simulink model "gate_V37_2016a_HIL_C"  (R2016a)
settings: Solver=FixedStepDiscrete  StartTime=0.0  StopTime=120  FixedStep=0.001  RelTol=1e-3
values from neighbouring files (symbols this model uses):
  CLOSE_SIDE_VALUE = single(4*ONE_SEC)    [GateParameter.m]
  CLOSED = uint8(12)    [GateParameter.m]
  CLOSING = uint8(13)    [GateParameter.m]
  CURRENT_STABLE_TIMER = uint8(200)    [GateParameter.m]
  CURRENT_THRESH = uint16(1000)    [GateParameter.m]
  INIT = uint8(15)    [GateParameter.m]
  MOVING_TIMER = uint32(60*ONE_SEC)    [GateParameter.m]
  OPEN = uint8(14)    [GateParameter.m]
  OPEN_SIDE_VALUE = uint16(4*ONE_SEC)    [GateParameter.m]
  OPENING = uint8(11)    [GateParameter.m]
  OPENING_ACTIVE = uint8(1)    [GateParameter.m]
  STABLE_TIMER_VALUE = uint16(1*ONE_SEC)    [GateParameter.m]
undefined symbols (not defined in the file or in its folder): sigbuilder_block
libraries: simulink x9, arduinolib x7
(part 1 of 2)
settings Model: ExtModeTrigDuration=2  Creator=omaro  LastModifiedBy=oelkawok
settings DataIOCC: SaveFormat=StructureWithTime  SaveOutput=off  SaveTime=off
settings OptimizationCC: StateBitsets=on  DataBitsets=on  LifeSpan=1  MaxStackSize=64
settings DebuggingCC: ConsistencyChecking=error  SignalInfNanChecking=error  SignalRangeChecking=error  ReadBeforeWriteMsg=EnableAllAsError  WriteAfterWriteMsg=EnableAllAsError  WriteAfterReadMsg=EnableAllAsError  AlgebraicLoopMsg=error  ArtificialAlgebraicLoopMsg=error  BlockPriorityViolationMsg=error  MinStepSizeMsg=error  CheckMatrixSingularityMsg=error  ParameterUnderflowMsg=error  ParameterPrecisionLossMsg=error  UnderSpecifiedDataTypeMsg=warning  UnnecessaryDatatypeConvMsg=warning  VectorMatrixConversionMsg=error  SignalLabelMismatchMsg=error  UnconnectedInputMsg=error  UnconnectedOutputMsg=error  UnconnectedLineMsg=error  SFcnCompatibilityMsg=error  UniqueDataStoreMsg=error  BusObjectLabelMismatch=error  RootOutportRequireBusObject=error  ModelReferenceIOMsg=error  ModelReferenceVersionMismatchMessage=warning  ModelReferenceIOMismatchMessage=error  ModelReferenceDataLoggingMessage=error  StrictBusMsg=ErrorOnBusTreatedAsVector  SFUnusedDataAndEventsDiag=error  SFUnexpectedBacktrackingDiag=error  SFInvalidInputDataAccessInChartInitDiag=error  SFNoUnconditionalDefaultTransitionDiag=error  SFTransitionOutsideNaturalParentDiag=error  SFUndirectedBroadcastEventsDiag=error  SFTransitionActionBeforeConditionDiag=error  SFOutputUsedAsStateInMooreChartDiag=warning
settings HardwareCC: ProdBitPerInt=16  ProdBitPerPointer=16  ProdLargestAtomicFloat=None  ProdWordSize=8  ProdHWDeviceType=Atmel->AVR
settings SFSimCC: SimCustomHeaderCode=|
  | //#include "C:\Users\omaro\Desktop\Gate_Model\Arduino\Arduino.h"
  | //#include "Keypad\Keypad.h"
settings RTWCC: SystemTargetFile=ert.tlc  HardwareBoard=Arduino Nano 3.0  TemplateMakefile=ert_default_tmf  GenerateReport=on  RTWUseSimCustomCode=on  Toolchain=Arduino AVR v1.6.1  BuildConfiguration=Faster Runs  LaunchReport=on
settings RTWCC: CustomHeaderCode=|
  | //#include "C:\Users\omaro\Desktop\Gate_Model\Arduino\Arduino.h"
  | //#include "Keypad\Keypad.h"
settings CodeAppCC: ForceParamTrailComments=on  OperatorAnnotations=on  MATLABSourceComments=on
settings ERTTargetCC: ERTCustomFileBanners=off  SupportContinuousTime=on  SupportNonInlinedSFcns=on  SupportVariableSizeSignals=on  ModelStepFunctionPrototypeControlCompliant=off  RootIOFormat=Structure reference
settings ConfigComp: CovEnable=on  CovScope=ReferencedModels  CovIncludeTopModel=off  CovPath=Gate  CovMetricSettings=dcw  CovHTMLOptions=-sRT=1 -sVT=0 -aTS=1 -bRG=1 -bTC=1 -hTR=0 -nFC=0 -nFD=1 -scm=1 -bcm=1 -xEv=1 -xEM=0  CovHtmlReporting=off  CovModelRefEnable=all

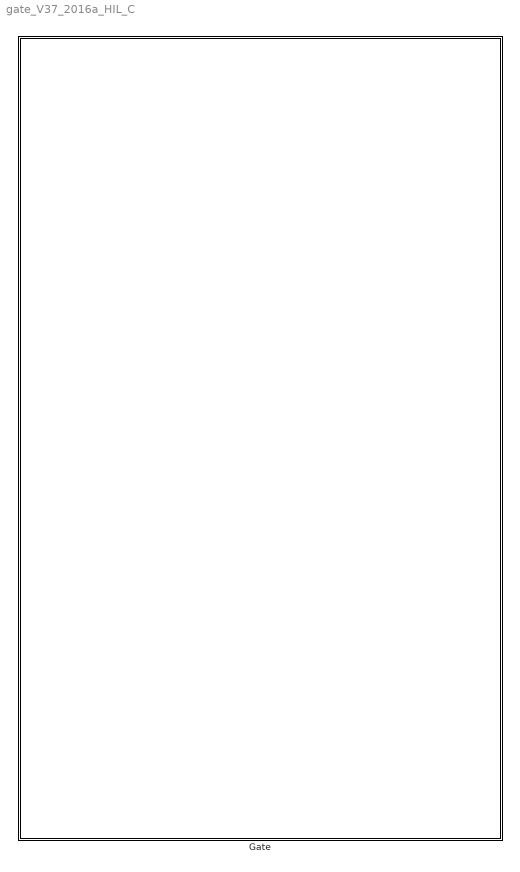
== system gate_V37_2016a_HIL_C ==
  Gate : Subsystem

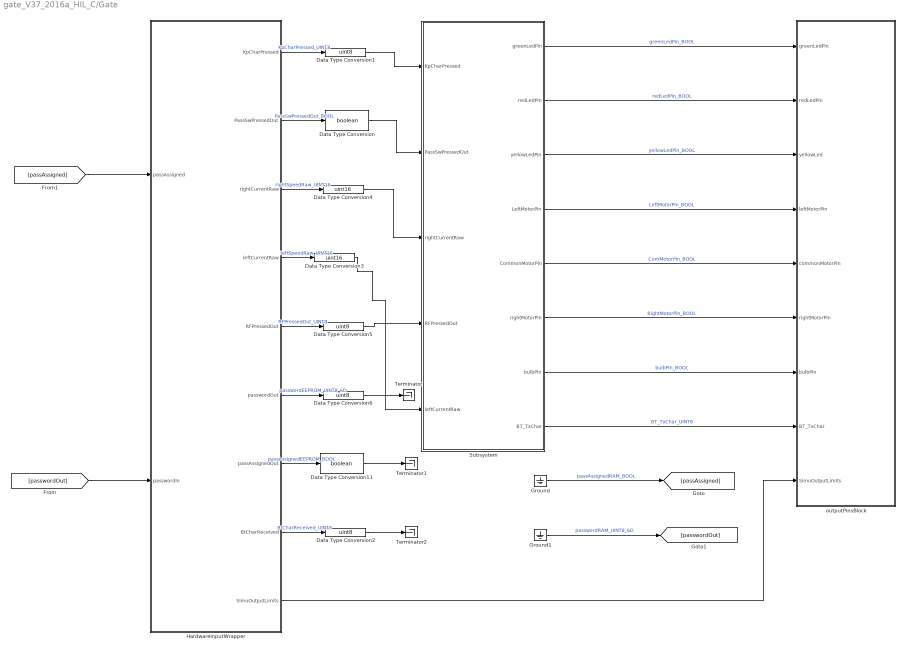
== system gate_V37_2016a_HIL_C/Gate ==
  Data Type Conversion : DataTypeConversion  OutDataTypeStr=boolean
  Data Type Conversion{1..2,5..6} (4 alike) : DataTypeConversion  OutDataTypeStr=uint8
  Data Type Conversion11 : DataTypeConversion  OutDataTypeStr=boolean
  Data Type Conversion3 : DataTypeConversion  OutDataTypeStr=uint16
  Data Type Conversion4 : DataTypeConversion  OutDataTypeStr=uint16
  Ground : Ground
  Ground1 : Ground
  HardwareInputWrapper : Subsystem  Variant=on  VariantControl=Variant1  OverrideUsingVariant=HardwareInloopInputs_C
  Subsystem : Subsystem
  Terminator : Terminator
  Terminator1 : Terminator
  Terminator2 : Terminator
  outputPinsBlock : Subsystem  Variant=on  VariantControl=Variant1  OverrideUsingVariant=HardwareInLoopOutputs_C
  wires:
  HardwareInputWrapper/9(SimuOutputLimits) -> outputPinsBlock/9(SimuOutputLimits)
  HardwareInputWrapper/8(BtCharReceived) -> Data Type Conversion2/1  "BtCharReceived_UINT8"
  HardwareInputWrapper/2(PassSwPressedOut) -> Data Type Conversion/1  "PassSwPressedOut_BOOL"
  HardwareInputWrapper/3(rightCurrentRaw) -> Data Type Conversion4/1  "rightSpeedRaw_UINT16"
  HardwareInputWrapper/5(RFPressedOut) -> Data Type Conversion5/1  "RFPressedOut_UINT8"
  Subsystem/1(greenLedPin) -> outputPinsBlock/1(greenLedPin)  "greenLedPin_BOOL"
  Subsystem/2(redLedPin) -> outputPinsBlock/2(redLedPin)  "redLedPin_BOOL"
  Subsystem/3(yellowLedPin) -> outputPinsBlock/3(yellowLed)  "yellowLedPin_BOOL"
  Subsystem/4(LeftMotorPin) -> outputPinsBlock/4(leftMotorPin)  "LeftMotorPin_BOOL"
  Subsystem/5(CommonMotorPin) -> outputPinsBlock/5(commonMotorPin)  "ComMotorPin_BOOL"
  Subsystem/6(rightMotorPin) -> outputPinsBlock/6(rightMotorPin)  "RightMotorPin_BOOL"
  Subsystem/7(bulbPin) -> outputPinsBlock/7(bulbPin)  "bulbPin_BOOL"
  Subsystem/8(BT_TxChar) -> outputPinsBlock/8(BT_TxChar)  "BT_TxChar_UINT8"
  HardwareInputWrapper/4(leftCurrentRaw) -> Data Type Conversion3/1  "leftSpeedRaw_UINT16"
  HardwareInputWrapper/1(KpCharPressed) -> Data Type Conversion1/1  "KpCharPressed_UINT8"
  Ground1/1 -> [passwordOut]  "passwordRAM_UINT8_6D"
  Ground/1 -> [passAssigned]  "passAssignedRAM_BOOL"
  [passwordOut] -> HardwareInputWrapper/2(passwordIn)
  [passAssigned] -> HardwareInputWrapper/1(passAssigned)
  HardwareInputWrapper/6(passwordOut) -> Data Type Conversion6/1  "passwordEEPROM_UINT8_6D"
  HardwareInputWrapper/7(passAssignedOut) -> Data Type Conversion11/1  "passAssignedEEPROM_BOOL"
  Data Type Conversion1/1 -> Subsystem/1(KpCharPressed)
  Data Type Conversion/1 -> Subsystem/2(PassSwPressedOut)
  Data Type Conversion4/1 -> Subsystem/3(rightCurrentRaw)
  Data Type Conversion3/1 -> Subsystem/5(leftCurrentRaw)
  Data Type Conversion5/1 -> Subsystem/4(RFPressedOut)
  Data Type Conversion11/1 -> Terminator1/1
  Data Type Conversion6/1 -> Terminator/1
  Data Type Conversion2/1 -> Terminator2/1

== system gate_V37_2016a_HIL_C/Gate/HardwareInputWrapper ==
  passAssigned : Inport
  passwordIn : Inport  Port=2
  HIL_B : Subsystem  VariantControl=HardwareInloopInputs_B
  HIL_C : Subsystem  VariantControl=HardwareInloopInputs_C
  MIL_Gate_A : Subsystem  VariantControl=MIL_VariantA
  MIL_Gate_B : Subsystem  VariantControl=MIL_VariantB
  MIL_Gate_C : Subsystem  VariantControl=MIL_VariantC
  KpCharPressed : Outport
  PassSwPressedOut : Outport  Port=2
  rightCurrentRaw : Outport  Port=3
  leftCurrentRaw : Outport  Port=4
  RFPressedOut : Outport  Port=5
  passwordOut : Outport  Port=6
  passAssignedOut : Outport  Port=7
  BtCharReceived : Outport  Port=8
  SimuOutputLimits : Outport  Port=9

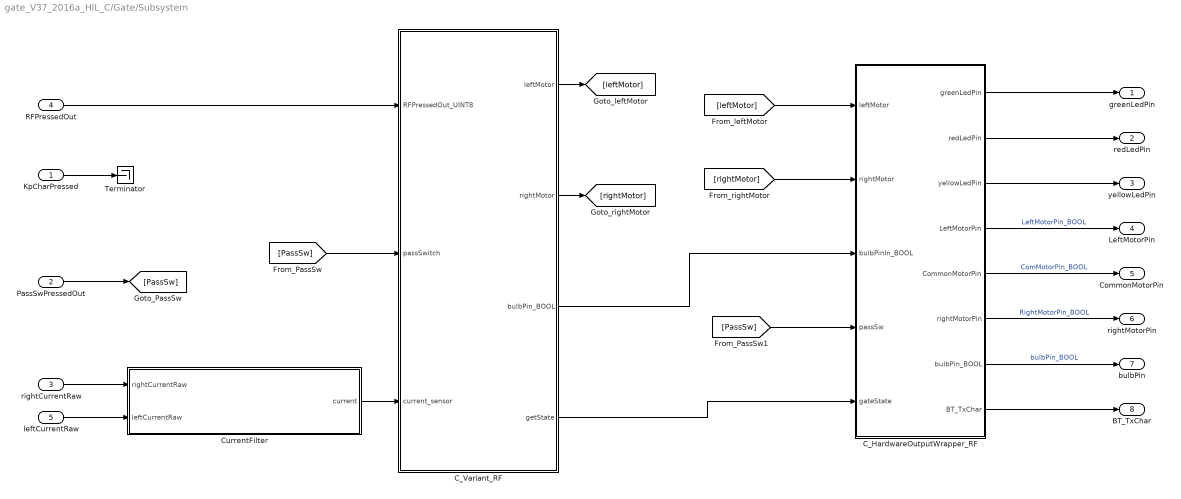
== system gate_V37_2016a_HIL_C/Gate/Subsystem ==
  KpCharPressed : Inport  OutDataTypeStr=uint8  PortDimensions=1  SampleTime=0
  PassSwPressedOut : Inport  Port=2  OutDataTypeStr=boolean  PortDimensions=1  SampleTime=0
  rightCurrentRaw : Inport  Port=3  OutDataTypeStr=uint16  PortDimensions=1  SampleTime=0
  RFPressedOut : Inport  Port=4  OutDataTypeStr=uint8  PortDimensions=1  SampleTime=0
  leftCurrentRaw : Inport  Port=5  OutDataTypeStr=uint16  PortDimensions=1  SampleTime=0
  C_HardwareOutputWrapper_RF : Subsystem  VariantControl=Variant3
  C_Variant_RF : Subsystem  VariantControl=Variant3
  CurrentFilter : Subsystem
  Terminator : Terminator
  greenLedPin : Outport  OutDataTypeStr=double  PortDimensions=1  SampleTime=[0.001,0]
  redLedPin : Outport  Port=2  OutDataTypeStr=double  PortDimensions=1  SampleTime=[0.001,0]
  yellowLedPin : Outport  Port=3  OutDataTypeStr=double  PortDimensions=1  SampleTime=[0.001,0]
  LeftMotorPin : Outport  Port=4  OutDataTypeStr=boolean  PortDimensions=1  SampleTime=[0.001,0]
  CommonMotorPin : Outport  Port=5  OutDataTypeStr=boolean  PortDimensions=1  SampleTime=[0.001,0]
  rightMotorPin : Outport  Port=6  OutDataTypeStr=boolean  PortDimensions=1  SampleTime=[0.001,0]
  bulbPin : Outport  Port=7  OutDataTypeStr=boolean  PortDimensions=1  SampleTime=[0.001,0]
  BT_TxChar : Outport  Port=8  OutDataTypeStr=double  PortDimensions=1  SampleTime=[0.001,0]
  wires:
  PassSwPressedOut/1 -> [PassSw]
  KpCharPressed/1 -> Terminator/1
  [PassSw] -> C_Variant_RF/2(passSwitch)
  rightCurrentRaw/1 -> CurrentFilter/1(rightCurrentRaw)
  C_Variant_RF/1(leftMotor) -> [leftMotor]
  C_Variant_RF/2(rightMotor) -> [rightMotor]
  [leftMotor] -> C_HardwareOutputWrapper_RF/1(leftMotor)
  [rightMotor] -> C_HardwareOutputWrapper_RF/2(rightMotor)
  C_Variant_RF/3(bulbPin_BOOL) -> C_HardwareOutputWrapper_RF/3(bulbPinIn_BOOL)
  [PassSw] -> C_HardwareOutputWrapper_RF/4(passSw)
  C_HardwareOutputWrapper_RF/1(greenLedPin) -> greenLedPin/1
  C_HardwareOutputWrapper_RF/2(redLedPin) -> redLedPin/1
  C_HardwareOutputWrapper_RF/3(yellowLedPin) -> yellowLedPin/1
  C_HardwareOutputWrapper_RF/4(LeftMotorPin) -> LeftMotorPin/1  "LeftMotorPin_BOOL"
  C_HardwareOutputWrapper_RF/5(CommonMotorPin) -> CommonMotorPin/1  "ComMotorPin_BOOL"
  C_HardwareOutputWrapper_RF/6(rightMotorPin) -> rightMotorPin/1  "RightMotorPin_BOOL"
  C_HardwareOutputWrapper_RF/7(bulbPin_BOOL) -> bulbPin/1  "bulbPin_BOOL"
  C_HardwareOutputWrapper_RF/8(BT_TxChar) -> BT_TxChar/1
  CurrentFilter/1(current) -> C_Variant_RF/3(current_sensor)
  leftCurrentRaw/1 -> CurrentFilter/2(leftCurrentRaw)
  RFPressedOut/1 -> C_Variant_RF/1(RFPressedOut_UINT8)
  C_Variant_RF/4(getState) -> C_HardwareOutputWrapper_RF/5(gateState)

== system gate_V37_2016a_HIL_C/Gate/outputPinsBlock ==
  greenLedPin : Inport
  redLedPin : Inport  Port=2
  yellowLed : Inport  Port=3
  leftMotorPin : Inport  Port=4
  commonMotorPin : Inport  Port=5
  rightMotorPin : Inport  Port=6
  bulbPin : Inport  Port=7
  BT_TxChar : Inport  Port=8
  SimuOutputLimits : Inport  Port=9
  HIL_B : Subsystem  VariantControl=HardwareInLoopOutputs_B
  HIL_C : Subsystem  VariantControl=HardwareInLoopOutputs_C
  MIL : Subsystem  Variant=on  VariantControl=MIL_ALL_Variants  OverrideUsingVariant=VVOutputs

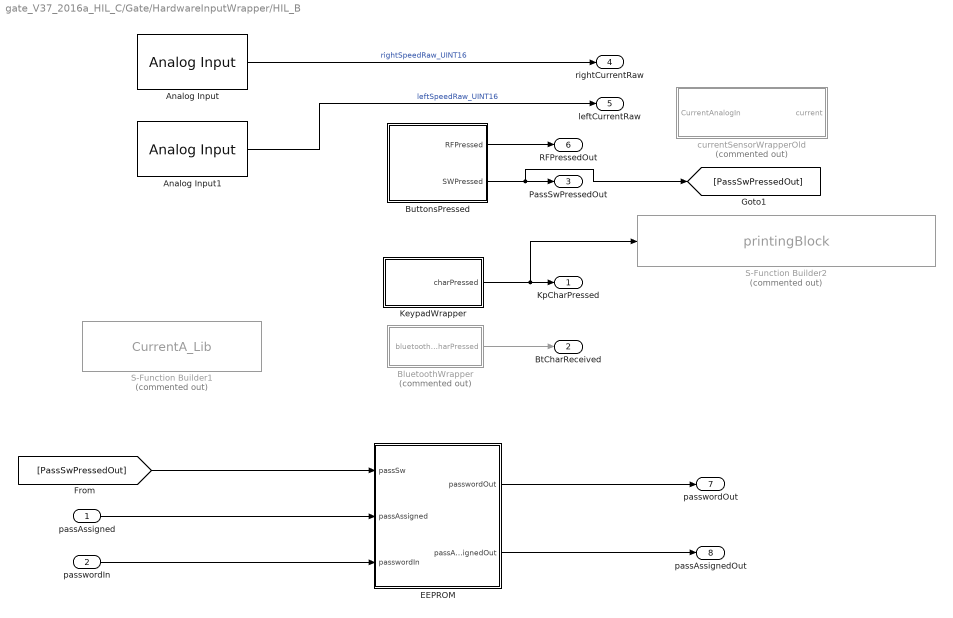
== system gate_V37_2016a_HIL_C/Gate/HardwareInputWrapper/HIL_B ==
  passAssigned : Inport
  passwordIn : Inport  Port=2
  Analog Input : arduinolib/Analog Input  pinNumber=6  sampleTime=-1  blockPlatform=arduino
  Analog Input1 : arduinolib/Analog Input  pinNumber=7  sampleTime=-1  blockPlatform=arduino
  BluetoothWrapper : Subsystem  Commented=on
  ButtonsPressed : Subsystem
  EEPROM : Subsystem
  KeypadWrapper : Subsystem
  S-Function Builder1 : S-Function  Commented=on  InitFcn=try, set_param(gcb,'FunctionName','CurrentA_Lib'), end  FunctionName=CurrentA_Lib  SFunctionModules=CurrentA_Lib_wrapper  SFunctionDeploymentMode=off  EnableBusSupport=off  mask(S-Function Builder){}
      mask help: Simulink:masks:SFunctionBuilder_MD
      mask init:
      | val = [[.9  0.75 0.75 1 1 .9 .9 1]',[1 1 0.75 0.75 .9 .9 1 .9]'];try , sys = get_param(gcb,'SfunBuilderFcnName');if isempty(sys), sys = get_param(gcb,'FunctionName'); end,catch, sys = get_param(gcb,'FunctionName'); end
      settings: CopyFcn=set_param(gcbh,'LinkStatus','none')  PreSaveFcn=try, set_param(gcb,'FunctionName','CurrentA_Lib'), end  OpenFcn=sfunctionwizard(gcbh)
      mask Display: plot(val(:,1),val(:,2)),disp(sys), port_label('input',1,'u0'), port_label('output',1,'y0')
  S-Function Builder2 : S-Function  Commented=on  InitFcn=try, set_param(gcb,'FunctionName','printingBlock'), end  FunctionName=printingBlock  SFunctionModules=printingBlock_wrapper  SFunctionDeploymentMode=off  EnableBusSupport=off  mask(S-Function Builder){}
      mask help: Simulink:masks:SFunctionBuilder_MD
      mask init: same as gate_V37_2016a_HIL_C/Gate/HardwareInputWrapper/HIL_B/S-Function Builder1
      settings: CopyFcn=set_param(gcbh,'LinkStatus','none')  PreSaveFcn=try, set_param(gcb,'FunctionName','printingBlock'), end  OpenFcn=sfunctionwizard(gcbh)
      mask Display: plot(val(:,1),val(:,2)),disp(sys), port_label('input',1,'u0'), port_label('output',1,'y0')
  currentSensorWrapperOld : Subsystem  Commented=on
  KpCharPressed : Outport
  BtCharReceived : Outport  Port=2
  PassSwPressedOut : Outport  Port=3
  rightCurrentRaw : Outport  Port=4
  leftCurrentRaw : Outport  Port=5
  RFPressedOut : Outport  Port=6
  passwordOut : Outport  Port=7
  passAssignedOut : Outport  Port=8
  wires:
  BluetoothWrapper/1(bluetoothCharPressed) -> BtCharReceived/1
  Analog Input/1 -> rightCurrentRaw/1  "rightSpeedRaw_UINT16"
  KeypadWrapper/1(charPressed) -> S-Function Builder2/1, KpCharPressed/1
  ButtonsPressed/2(SWPressed) -> [PassSwPressedOut], PassSwPressedOut/1
  ButtonsPressed/1(RFPressed) -> RFPressedOut/1
  Analog Input1/1 -> leftCurrentRaw/1  "leftSpeedRaw_UINT16"
  [PassSwPressedOut] -> EEPROM/1(passSw)
  passAssigned/1 -> EEPROM/2(passAssigned)
  passwordIn/1 -> EEPROM/3(passwordIn)
  EEPROM/1(passwordOut) -> passwordOut/1
  EEPROM/2(passAssignedOut) -> passAssignedOut/1

== system gate_V37_2016a_HIL_C/Gate/HardwareInputWrapper/HIL_C ==
  Analog Input : arduinolib/Analog Input  pinNumber=6  sampleTime=-1  blockPlatform=arduino
  Analog Input1 : arduinolib/Analog Input  pinNumber=7  sampleTime=-1  blockPlatform=arduino
  ButtonsPressed : Subsystem
  S-Function Builder2 : S-Function  Commented=on  InitFcn=try, set_param(gcb,'FunctionName','printingBlock'), end  FunctionName=printingBlock  SFunctionModules=printingBlock_wrapper  SFunctionDeploymentMode=off  EnableBusSupport=off  mask(S-Function Builder){}
      mask help: Simulink:masks:SFunctionBuilder_MD
      mask init: same as gate_V37_2016a_HIL_C/Gate/HardwareInputWrapper/HIL_B/S-Function Builder1
      settings: CopyFcn=set_param(gcbh,'LinkStatus','none')  PreSaveFcn=try, set_param(gcb,'FunctionName','printingBlock'), end  OpenFcn=sfunctionwizard(gcbh)
      mask Display: plot(val(:,1),val(:,2)),disp(sys), port_label('input',1,'u0'), port_label('output',1,'y0')
  PassSwPressedOut : Outport
  rightCurrentRaw : Outport  Port=2
  leftCurrentRaw : Outport  Port=3
  RFPressedOut : Outport  Port=4
  wires:
  Analog Input/1 -> rightCurrentRaw/1  "rightSpeedRaw_UINT16"
  ButtonsPressed/2(SWPressed) -> PassSwPressedOut/1
  ButtonsPressed/1(RFPressed) -> RFPressedOut/1
  Analog Input1/1 -> leftCurrentRaw/1  "leftSpeedRaw_UINT16"

== system gate_V37_2016a_HIL_C/Gate/HardwareInputWrapper/MIL_Gate_A ==
  Bus Creator : BusCreator  Inputs=16
  Mux : Mux  Inputs=6
  Quantizer{,1..3} (4 alike) : Quantizer  QuantizationInterval=-1
  Signal Builder : Subsystem  Tag=STV Subsys  LoadFcn=sigbuilder_block('load');  StartFcn=sigbuilder_block('start');  StopFcn=sigbuilder_block('stop');  ClipboardFcn=sigbuilder_block('clipboard');  mask(Sigbuilder block){}
      mask help: Simulink:masks:SigbuilderBlock_MD
      mask init:
      | if ~strcmp(get_param(bdroot(gcbh),'SimulationStatus'),'stopped') tuvar = sigbuilder_block('maskInit'); end
      settings: CopyFcn=sigbuilder_block('copy');  DeleteFcn=sigbuilder_block('delete');  ModelCloseFcn=sigbuilder_block('modelClose');  PreSaveFcn=sigbuilder_block('preSave');  PauseFcn=sigbuilder_block('pause');  ContinueFcn=sigbuilder_block('continue');  NameChangeFcn=sigbuilder_block('namechange');  OpenFcn=sigbuilder_block('open',[6 28.2 1012.8 484.8 ]);  CloseFcn=sigbuilder_block('close');
      mask Display: plot(0, 0, 100, 100,[2, 2, 32, 32, 2], [68, 8, 8, 68, 68],[32, 2], [38, 38], [32, 19, 2],[53, 60, 44], [32, 17, 17, 2],[16, 16, 31, 31]);txt = getActiveGroup(gcbh);text(2, 100, txt,'verticalAlignment', 'top');
  KpCharPressed : Outport
  BtCharReceived : Outport  Port=2
  PassSwPressedOut : Outport  Port=3
  RFPressedOut : Outport  Port=4
  rightCurrentRaw : Outport  Port=5
  leftCurrentRaw : Outport  Port=6
  SimuOutputLimits : Outport  Port=7
  passwordOut : Outport  Port=8
  passAssignedOut : Outport  Port=9
  wires:
  Signal Builder/2(BT_input) -> Quantizer1/1
  Signal Builder/4(SW_input) -> Quantizer3/1
  Signal Builder/1(Kp_input) -> Quantizer/1
  Signal Builder/3(RF_input) -> Quantizer2/1
  Quantizer/1 -> KpCharPressed/1
  Quantizer1/1 -> BtCharReceived/1
  Quantizer2/1 -> RFPressedOut/1
  Quantizer3/1 -> PassSwPressedOut/1
  Bus Creator/1 -> SimuOutputLimits/1
  Signal Builder/17(GreenLedMax) -> Bus Creator/11  "GreenLedMax"
  Signal Builder/16(YellowLedMin) -> Bus Creator/10  "YellowLedMin"
  Signal Builder/18(GreenLedMin) -> Bus Creator/12  "GreenLedMin"
  Signal Builder/15(YellowLedMax) -> Bus Creator/9  "YellowLedMax"
  Signal Builder/19(BulbMax) -> Bus Creator/13  "BulbMax"
  Signal Builder/14(RedLedMin) -> Bus Creator/8  "RedLedMin"
  Signal Builder/20(BulbMin) -> Bus Creator/14  "BulbMin"
  Signal Builder/21(BT_ouputMax) -> Bus Creator/15  "BT_outputMax"
  Signal Builder/13(RedLedMax) -> Bus Creator/7  "RedLedMax"
  Signal Builder/12(MCMin) -> Bus Creator/6  "MCMin"
  Signal Builder/11(MCMax) -> Bus Creator/5  "MCMax"
  Signal Builder/10(MRMin) -> Bus Creator/4  "MRMin"
  Signal Builder/9(MRMax) -> Bus Creator/3  "MRMax"
  Signal Builder/8(MLMin) -> Bus Creator/2  "MLMin"
  Signal Builder/7(MLMax) -> Bus Creator/1  "MLMax"
  Signal Builder/22(BT_outputMin) -> Bus Creator/16  "BT_outputMin"
  Signal Builder/5(rightSpeedRaw) -> rightCurrentRaw/1
  Signal Builder/6(leftSpeedRaw) -> leftCurrentRaw/1
  Signal Builder/23(PassAssignedEEPROM) -> passAssignedOut/1
  Mux/1 -> passwordOut/1
  Signal Builder/24(Pass1) -> Mux/1
  Signal Builder/25(Pass2) -> Mux/2
  Signal Builder/26(Pass3) -> Mux/3
  Signal Builder/27(Pass4) -> Mux/4
  Signal Builder/28(Pass5) -> Mux/5
  Signal Builder/29(Pass6) -> Mux/6

== system gate_V37_2016a_HIL_C/Gate/HardwareInputWrapper/MIL_Gate_B ==
  Bus Creator : BusCreator  Inputs=16
  Constant : Constant
  Constant1 : Constant  Value=-1
  Constant2 : Constant  Value=0
  Mux : Mux  Inputs=6
  Signal Builder : Subsystem  Tag=STV Subsys  LoadFcn=sigbuilder_block('load');  StartFcn=sigbuilder_block('start');  StopFcn=sigbuilder_block('stop');  ClipboardFcn=sigbuilder_block('clipboard');  mask(Sigbuilder block){}
      mask help: Simulink:masks:SigbuilderBlock_MD
      mask init:
      | if ~strcmp(get_param(bdroot(gcbh),'SimulationStatus'),'stopped') tuvar = sigbuilder_block('maskInit'); end
      settings: CopyFcn=sigbuilder_block('copy');  DeleteFcn=sigbuilder_block('delete');  ModelCloseFcn=sigbuilder_block('modelClose');  PreSaveFcn=sigbuilder_block('preSave');  PauseFcn=sigbuilder_block('pause');  ContinueFcn=sigbuilder_block('continue');  NameChangeFcn=sigbuilder_block('namechange');  OpenFcn=sigbuilder_block('open',[5.4 33.6 1141.2 553.8 ]);  CloseFcn=sigbuilder_block('close');
      mask Display: plot(0, 0, 100, 100,[2, 2, 32, 32, 2], [68, 8, 8, 68, 68],[32, 2], [38, 38], [32, 19, 2],[53, 60, 44], [32, 17, 17, 2],[16, 16, 31, 31]);txt = getActiveGroup(gcbh);text(2, 100, txt,'verticalAlignment', 'top');
  Signal Builder1 : Subsystem  Tag=STV Subsys  Commented=on  LoadFcn=sigbuilder_block('load');  StartFcn=sigbuilder_block('start');  StopFcn=sigbuilder_block('stop');  ClipboardFcn=sigbuilder_block('clipboard');  mask(Sigbuilder block){}
      mask help: Simulink:masks:SigbuilderBlock_MD
      mask init:
      | if ~strcmp(get_param(bdroot(gcbh),'SimulationStatus'),'stopped') tuvar = sigbuilder_block('maskInit'); end
      settings: CopyFcn=sigbuilder_block('copy');  DeleteFcn=sigbuilder_block('delete');  ModelCloseFcn=sigbuilder_block('modelClose');  PreSaveFcn=sigbuilder_block('preSave');  PauseFcn=sigbuilder_block('pause');  ContinueFcn=sigbuilder_block('continue');  NameChangeFcn=sigbuilder_block('namechange');  OpenFcn=sigbuilder_block('open',[-1139.4 30 1012.8 507 ]);  CloseFcn=sigbuilder_block('close');
      mask Display: plot(0, 0, 100, 100,[2, 2, 32, 32, 2], [68, 8, 8, 68, 68],[32, 2], [38, 38], [32, 19, 2],[53, 60, 44], [32, 17, 17, 2],[16, 16, 31, 31]);txt = getActiveGroup(gcbh);text(2, 100, txt,'verticalAlignment', 'top');
  KpCharPressed : Outport
  PassSwPressedOut : Outport  Port=2
  RFPressedOut : Outport  Port=3
  rightCurrentRaw : Outport  Port=4
  leftCurrentRaw : Outport  Port=5
  SimuOutputLimits : Outport  Port=6
  BtCharReceived : Outport  Port=7
  passwordOut : Outport  Port=8
  passAssignedOut : Outport  Port=9
  wires:
  Signal Builder/6(MLMax) -> Bus Creator/1  "MLMax"
  Signal Builder/7(MLMin) -> Bus Creator/2  "MLMin"
  Signal Builder/8(MRMax) -> Bus Creator/3  "MRMax"
  Signal Builder/9(MRMin) -> Bus Creator/4  "MRMin"
  Signal Builder/10(MCMax) -> Bus Creator/5  "MCMax"
  Signal Builder/11(MCMin) -> Bus Creator/6  "MCMin"
  Signal Builder/12(RedLedMax) -> Bus Creator/7  "RedLedMax"
  Signal Builder/19(BulbMin) -> Bus Creator/14  "BulbMin"
  Signal Builder/13(RedLedMin) -> Bus Creator/8  "RedLedMin"
  Signal Builder/18(BulbMax) -> Bus Creator/13  "BulbMax"
  Signal Builder/14(YellowLedMax) -> Bus Creator/9  "YellowLedMax"
  Signal Builder/17(GreenLedMin) -> Bus Creator/12  "GreenLedMin"
  Signal Builder/15(YellowLedMin) -> Bus Creator/10  "YellowLedMin"
  Signal Builder/16(GreenLedMax) -> Bus Creator/11  "GreenLedMax"
  Bus Creator/1 -> SimuOutputLimits/1
  Constant/1 -> Bus Creator/15  "BT_outputMax"
  Constant1/1 -> Bus Creator/16  "BT_outputMin"
  Constant2/1 -> BtCharReceived/1
  Signal Builder/3(SW_input) -> PassSwPressedOut/1
  Mux/1 -> passwordOut/1
  Signal Builder/1(Kp_input) -> KpCharPressed/1
  Signal Builder/2(RF_input) -> RFPressedOut/1
  Signal Builder/4(rightSpeedRaw) -> rightCurrentRaw/1
  Signal Builder/5(leftSpeedRaw) -> leftCurrentRaw/1
  Signal Builder/21(Pass1) -> Mux/1
  Signal Builder/22(Pass2) -> Mux/2
  Signal Builder/23(Pass3) -> Mux/3
  Signal Builder/24(Pass4) -> Mux/4
  Signal Builder/25(Pass5) -> Mux/5
  Signal Builder/26(Pass6) -> Mux/6
  Signal Builder/20(PassAssignedEEPROM) -> passAssignedOut/1

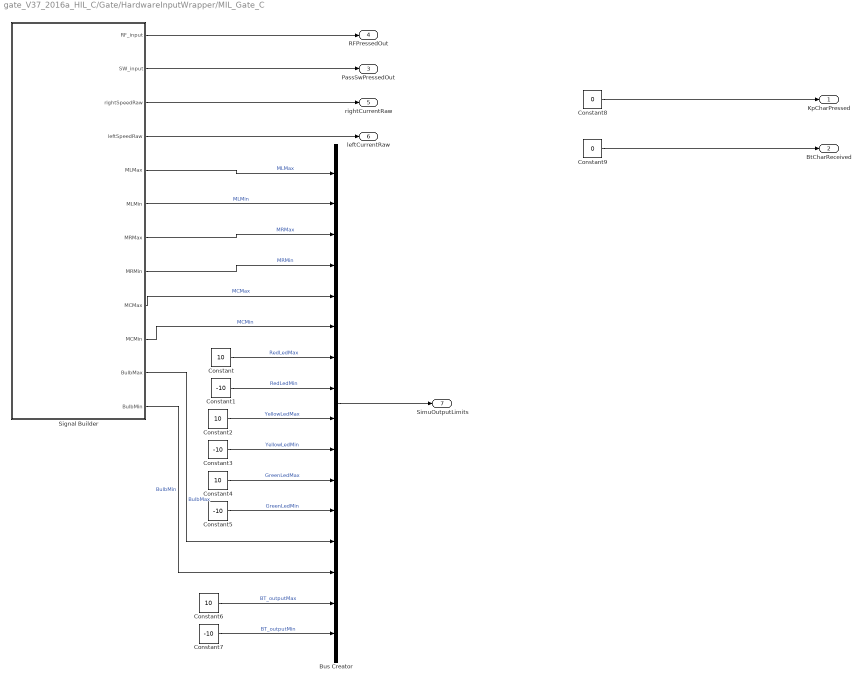
== system gate_V37_2016a_HIL_C/Gate/HardwareInputWrapper/MIL_Gate_C ==
  Bus Creator : BusCreator  Inputs=16
  Constant{,2,4,6} (4 alike) : Constant  Value=10
  Constant{1,3,5,7} (4 alike) : Constant  Value=-10
  Constant8 : Constant  Value=0
  Constant9 : Constant  Value=0
  Signal Builder : Subsystem  Tag=STV Subsys  LoadFcn=sigbuilder_block('load',[39 57.6 1012.8 507 ]);  StartFcn=sigbuilder_block('start');  StopFcn=sigbuilder_block('stop');  ClipboardFcn=sigbuilder_block('clipboard');  mask(Sigbuilder block){}
      mask help: Simulink:masks:SigbuilderBlock_MD
      mask init:
      | if ~strcmp(get_param(bdroot(gcbh),'SimulationStatus'),'stopped') tuvar = sigbuilder_block('maskInit'); end
      settings: CopyFcn=sigbuilder_block('copy');  DeleteFcn=sigbuilder_block('delete');  ModelCloseFcn=sigbuilder_block('modelClose');  PreSaveFcn=sigbuilder_block('preSave');  PauseFcn=sigbuilder_block('pause');  ContinueFcn=sigbuilder_block('continue');  NameChangeFcn=sigbuilder_block('namechange');  OpenFcn=sigbuilder_block('open',[6 6 1012.5 507 ]);  CloseFcn=sigbuilder_block('close');
      mask Display: plot(0, 0, 100, 100,[2, 2, 32, 32, 2], [68, 8, 8, 68, 68],[32, 2], [38, 38], [32, 19, 2],[53, 60, 44], [32, 17, 17, 2],[16, 16, 31, 31]);txt = getActiveGroup(gcbh);text(2, 100, txt,'verticalAlignment', 'top');
  KpCharPressed : Outport
  BtCharReceived : Outport  Port=2
  PassSwPressedOut : Outport  Port=3
  RFPressedOut : Outport  Port=4
  rightCurrentRaw : Outport  Port=5
  leftCurrentRaw : Outport  Port=6
  SimuOutputLimits : Outport  Port=7
  wires:
  Constant8/1 -> KpCharPressed/1
  Constant9/1 -> BtCharReceived/1
  Signal Builder/1(RF_input) -> RFPressedOut/1
  Signal Builder/2(SW_input) -> PassSwPressedOut/1
  Bus Creator/1 -> SimuOutputLimits/1
  Constant4/1 -> Bus Creator/11  "GreenLedMax"